simulink library "Renault_Library"  (Simulink 9.2)
settings: Solver=ode3  StartTime=0.0  StopTime=10.0  FixedStep=0.020  RelTol=1e-3  EnableMultiTasking=on
undefined symbols (not defined in the file or in its folder): ABC, AssystemProposal, b, chkval, CoeffA_C, CoeffB_C, CoeffMean_C, ConfTime_C, DiagDelay_C, DividePercent, FailureConfActive_C, FallingEdgeDuration_C, HighLevelThresholdVoltage_C, HighLevelVoltage_C, HighLevelVoltageTunable_C, InitValue_C, InputVoltRangeMax_C, InputVoltRangeMin_C, LowActiveContact_C, LowLevelThresholdVoltage_C, LowLevelVoltage_C, MaxOperatingVoltage_C, MinOperatingVoltage_C, mV2V_conversion, OUT1, OutOfRangeHigh_C, OutOfRangeLow_C, PinSupplySaturationMax, PinSupplySaturationMin, Res1Value_C, Res2Value_C, Res3Value_C, RisingEdgeDuration_C, RSA_CANSignalFailMonitoring, SampleTime_C, service14Table, service19Table, service22Table, service24Table, service28Table, service2ETable, service2FTable, service31Table, service87Table, SizeXStateDID2FTable, SizeXTable14, SizeXTable19, SizeXTable22, SizeXTable24, SizeXTable28, SizeXTable2E, SizeXTable2F, SizeXTable31, SizeXTable87, SizeYStateDID2FTable, SizeYTable14, SizeYTable19, SizeYTable22, SizeYTable24, SizeYTable28 ... (+22)
undefined symbols (not defined in the file): BatteryVoltage_ActivationThreshold_C, FrameStatus_AbsentValue_C, FrameStatus_ConfAbsentValue_C, MemThreshold_C, NAMEOFTHEMODEL_SAMPLE_TIME_CST, NbAbs1_C, NbConfAbs1_C, NbConfPres1_C, NbPres1_C, NOMDUSIGNAL_FaultConfTime_C, NOMDUSIGNAL_FaultRehabTime_C, NOMDUSIGNAL_switchvalue_C, NOMDUSIGNAL_unavailablevalue_C, PeriodFrame1_C, ResetTime_C, WakeUpTime_C
libraries: $bdroot x112, simulink x75
(part 7 of 32)

== system Renault_Library/Services ECU/HW Outputs ==
  note: DEFINED OUTPUTS
  note: FULLY TUNABLE OUTPUTS
  RSA_IHAB6Y : Subsystem  Tag=[LIB]  mask(RSA_IHAB6Y){LowLevelVoltage_C=500, InitValue_C=0, RisingEdgeDuration_C=0, FallingEdgeDuration_C=0, SampleTime_C=1, Tstab_C=30, Res1Value_C=100000, Res2Value_C=50000, Res3Value_C=50000}
      mask prompts: LowLevelVoltage_C "Low Level Voltage (mV)"; InitValue_C "Initial value of Output"; RisingEdgeDuration_C "Duration of the rising edge (ms)"; FallingEdgeDuration_C "Duration of the falling edge (ms)"; SampleTime_C "SampleTime : insert a sample time (ms)"; Tstab_C "Tstab : insert a stabilization waiting time (ms)"; Res1Value_C "Resistor 1 Value (Ohm)"; Res2Value_C "Resisitor 2 Value (Ohm)"; Res3Value_C "Resistor 3 Value (Ohm)"
      mask help:
      | IHAB.6.Y: High side Open collector (Y= 25 or 45mA)
      | Min Operating Voltage = 6 V
      | Max Operating Voltage = 16 V
      | High Level voltage = SupplyVoltage
      | Low Level Voltage = "to be parameterized"
      | Unit of ports for voltage are in millivolt (mV)
      |
      | Version : 01.01
      mask init:
      | MinOperatingVoltage_C=6000;
      | MaxOperatingVoltage_C= 16000; 
      | LowActiveContact_C=0; %1= LowActiveContact; 0= HighActiveContact
      | SampleTimeSec_C=SampleTime_C*.001; %unit conversion for sampletime
      mask Help:
      | IHAB.6.Y: High side Open collector (Y= 25 or 45mA)
      | Min Operating Voltage = 6 V
      | Max Operating Voltage = 16 V
      | High Level voltage = SupplyVoltage
      | Low Level Voltage = "to be parameterized"
      | Unit of ports for voltage are in millivolt (mV)
      | 
      | Version : 01.01
      mask Display: disp('IHAB_6_Y')
  RSA_IHABD6Y : Subsystem  Tag=[LIB]  mask(RSA_IHABD6Y){LowLevelVoltage_C=500, InitValue_C=0, RisingEdgeDuration_C=0, FallingEdgeDuration_C=0, SampleTime_C=1, Tstab_C=30, ConfTime_C=20, DiagDelay_C=30, VoffMaxUser_C=6000, VoffMinUser_C=1000, VonMaxUser_C=16000, VonMinUser_C=5000, Res1Value_C=100000, Res2Value_C=50000, Res3Value_C=50000}
      mask prompts: LowLevelVoltage_C "Low Level Voltage (mV)"; InitValue_C "Initial Value of Output"; RisingEdgeDuration_C "Duration of the rising edge (ms)"; FallingEdgeDuration_C "Duration of the falling edge (ms)"; SampleTime_C "SampleTime : insert a sample time (ms)"; Tstab_C "Tstab : insert a stabilization waiting time (ms)"; ConfTime_C "ConfTime: Fault Confirmation time (ms)"; DiagDelay_C "DiagDelay: Delay in (ms) to take into account input state"; VoffMaxUser_C "Threshold (mV) above which an SC+ failure is declared when T is OFF : Voff_max"; VoffMinUser_C "Threshold (mV) below which a RES SC- failure is declared when T is OFF: Voff_min"; VonMaxUser_C "Threshold (mV) above which an RES SC-/ SC+ failure is declared when T is ON : Von_max"; VonMinUser_C "Threshold (mV) below which an SC- failure is declared when T is ON :Von_min"; Res1Value_C "Resistor 1 Value (Ohm)"; Res2Value_C "Resisitor 2 Value (Ohm)"; Res3Value_C "Resistor 3 Value (Ohm)"
      mask help:
      | IHAB.D.6.Y: High side Open collector with OC diagnosis capability(Y=25 or 45mA)
      |
      | ECU output, active on high state. Link to one producer.
      | Min Operating Voltage = 6 V
      | Max Operating Voltage = 16 V
      | (Supply Voltage - coeff) < High Level voltage < SupplyVoltage
      | Low Level Voltage = "to be parametered"
      | Unit of ports for voltage are in millivolt (mV)
      |
      | 0.8V < Coeff < 2V following the value of SupplyVoltage
      |
      | Diagnosis:
      | Three failure states (SC+, SC-, OC), and two normal states (OKON and OKOFF) are possible.
      | The analogue thresholds to output these different states are tunable in diagnostic Parameters tab.
      |
      |    State Value      Diag voltage         Diagnostic States
      |      5              V > Voffmax                 SC+
      |      4         Voffmax > V > Voffmin            OKOFF
      |      3         Voffmin > V > Vonmax         Resistive SC-
      |      2          Vonmax > V > Vonmin             OKON
      |      1              V < Vonmin                  SC-
      |
      | Version : 01.01
      mask init:
      | MinOperatingVoltage_C= 6000;
      | MaxOperatingVoltage_C= 16000; 
      | LowActiveContact_C=0; %1= LowActiveContact; 0= HighActiveContact
      | SampleTimeSec_C=SampleTime_C*.001; %unit conversion for sampletime
      | FailureConfActive_C=1; %to enable the confirmation bloc (otherwise output remains to 0)
      | State_InfVonMin_C=1;
      | State_Von_C=2;
      | State_SupVonMax_C=3;
      | State_InfVoffMin_C=3;
      | State_Voff_C=4;
      | State_SupVoffMax_C=5;
      | CoeffCorr = Res3Value_C/(Res2Value_C+Res3Value_C);
      | VoffMax_C = VoffMaxUser_C*CoeffCorr;
      | VoffMin_C = VoffMinUser_C*CoeffCorr;
      | VonMax_C = VonMaxUser_C*CoeffCorr;
      | VonMin_C = VonMinUser_C*CoeffCorr;
      mask Help:
      | IHAB.D.6.Y: High side Open collector with OC diagnosis capability(Y=25 or 45mA)
      | 
      | ECU output, active on high state. Link to one producer.
      | Min Operating Voltage = 6 V
      | Max Operating Voltage = 16 V
      | (Supply Voltage - coeff) < High Level voltage < SupplyVoltage
      | Low Level Voltage = "to be parametered"
      | Unit of ports for voltage are in millivolt (mV)
      | 
      | 0.8V < Coeff < 2V following the value of SupplyVoltage
      | 
      | Diagnosis:
      | Three failure states (SC+, SC-, OC), and two normal states (OKON and OKOFF) are possible.
      | The analogue thresholds to output these different states are tunable in diagnostic Parameters tab.
      | 
      |    State Value      Diag voltage         Diagnostic States
      |      5              V > Voffmax                 SC+
      |      4         Voffmax > V > Voffmin            OKOFF
      |      3         Voffmin > V > Vonmax         Resistive SC-
      |      2          Vonmax > V > Vonmin             OKON
      |      1              V < Vonmin                  SC-
      | 
      | Version : 01.01
      mask Display: disp('IHAB_D_6_Y')
  RSA_IHABDXY : Subsystem  Tag=[LIB]  mask(RSA_IHABDXY){MinOperatingVoltage_C=0, MaxOperatingVoltage_C=0, LowLevelVoltage_C=500, InitValue_C=0, RisingEdgeDuration_C=0, FallingEdgeDuration_C=0, SampleTime_C=1, Tstab_C=30, ConfTime_C=20, DiagDelay_C=30, VoffMaxUser_C=6000, VoffMinUser_C=1000, VonMaxUser_C=16000, VonMinUser_C=5000, Res1Value_C=100000, Res2Value_C=50000, Res3Value_C=50000}
      mask prompts: MinOperatingVoltage_C "Min Operating Voltage (mV)"; MaxOperatingVoltage_C "Max Operating Voltage (mV)"; LowLevelVoltage_C "Low Level Voltage (mV)"; InitValue_C "Initial Value of Output"; RisingEdgeDuration_C "Duration of the rising edge (ms)"; FallingEdgeDuration_C "Duration of the falling edge (ms)"; SampleTime_C "SampleTime : insert a sample time (ms)"; Tstab_C "Tstab : insert a stabilization waiting time (ms)"; ConfTime_C "ConfTime: Fault Confirmation time (ms)"; DiagDelay_C "DiagDelay: Delay in (ms) to take into account input state"; VoffMaxUser_C "Threshold (mV) above which an SC+ failure is declared when T is OFF : Voff_max"; VoffMinUser_C "Threshold (mV) below which a RES SC- failure is declared when T is OFF: Voff_min"; VonMaxUser_C "Threshold (mV) above which an RES SC-/ SC+ failure is declared when T is ON : Von_max"; VonMinUser_C "Threshold (mV) below which an SC- failure is declared when T is ON :Von_min"; Res1Value_C "Resistor 1 Value (Ohm)"; Res2Value_C "Resisitor 2 Value (Ohm)"; Res3Value_C "Resistor 3 Value (Ohm)"
      mask help:
      | IHAB.D.X.Y: High side Open collector with OC diagnosis capability(Y=25 or 45mA)
      |
      | ECU output, active on high state. Link to one producer.
      | Working with a minimum supply voltage of X V.
      | (Supply Voltage - coeff) < High Level voltage < SupplyVoltage
      | Low Level Voltage = "to be parametered"
      | Unit of ports for voltage are in millivolt (mV)
      |
      | 0.8V < Coeff < 2V following the value of SupplyVoltage
      |
      | Diagnosis:
      | Three failure states (SC+, SC-, OC), and two normal states (OKON and OKOFF) are possible.
      | The analogue thresholds to output these different states are tunable in diagnostic Parameters tab.
      |
      |    State Value      Diag voltage         Diagnostic States
      |      5              V > Voffmax                 SC+
      |      4         Voffmax > V > Voffmin            OKOFF
      |      3         Voffmin > V > Vonmax         Resistive SC-
      |      2          Vonmax > V > Vonmin             OKON
      |      1              V < Vonmin                  SC-
      |
      | Version : 01.01
      mask init:
      | LowActiveContact_C=0; %1= LowActiveContact; 0= HighActiveContact
      | SampleTimeSec_C=SampleTime_C*.001; %unit conversion for sampletime
      | FailureConfActive_C=1; %to enable the confirmation bloc (otherwise output remains to 0)
      | State_InfVonMin_C=1;
      | State_Von_C=2;
      | State_SupVonMax_C=3;
      | State_InfVoffMin_C=3;
      | State_Voff_C=4;
      | State_SupVoffMax_C=5;
      | CoeffCorr = Res3Value_C/(Res2Value_C+Res3Value_C);
      | VoffMax_C = VoffMaxUser_C*CoeffCorr;
      | VoffMin_C = VoffMinUser_C*CoeffCorr;
      | VonMax_C = VonMaxUser_C*CoeffCorr;
      | VonMin_C = VonMinUser_C*CoeffCorr;
      mask Help:
      | IHAB.D.X.Y: High side Open collector with OC diagnosis capability(Y=25 or 45mA)
      | 
      | ECU output, active on high state. Link to one producer.
      | Working with a minimum supply voltage of X V.
      | (Supply Voltage - coeff) < High Level voltage < SupplyVoltage
      | Low Level Voltage = "to be parametered"
      | Unit of ports for voltage are in millivolt (mV)
      | 
      | 0.8V < Coeff < 2V following the value of SupplyVoltage
      | 
      | Diagnosis:
      | Three failure states (SC+, SC-, OC), and two normal states (OKON and OKOFF) are possible.
      | The analogue thresholds to output these different states are tunable in diagnostic Parameters tab.
      | 
      |    State Value      Diag voltage         Diagnostic States
      |      5              V > Voffmax                 SC+
      |      4         Voffmax > V > Voffmin            OKOFF
      |      3         Voffmin > V > Vonmax         Resistive SC-
      |      2          Vonmax > V > Vonmin             OKON
      |      1              V < Vonmin                  SC-
      | 
      | Version : 01.01
      mask Display: disp('IHAB_D_X_Y')
  RSA_ILAB6Y : Subsystem  Tag=[LIB]  mask(RSA_ILAB6Y){LowLevelVoltage_C=500, InitValue_C=0, RisingEdgeDuration_C=0, FallingEdgeDuration_C=0, SampleTime_C=1, Tstab_C=30}
      mask prompts: LowLevelVoltage_C "Low Level Voltage (mV)"; InitValue_C "Initial Value of Output"; RisingEdgeDuration_C "Duration of the rising edge (ms)"; FallingEdgeDuration_C "Duration of the falling edge (ms)"; SampleTime_C "SampleTime : insert a sample time (ms)"; Tstab_C "Tstab : insert a stabilization waiting time (ms)"
      mask help:
      | ILAB.6.Y: Low side Open collector(Y=25 or 45mA)
      | Min Operating Voltage = 6 V
      | Max Operating Voltage = 16 V
      | High Level voltage = SupplyVoltage
      | Low Level Voltage = "to be parameterized"
      | Unit of ports for voltage are in millivolt (mV)
      |
      | Version : 01.01
      mask init:
      | MinOperatingVoltage_C=6000;
      | MaxOperatingVoltage_C= 16000;
      | HighLevelVoltage_C=0;
      | HighLevelVoltageTunable_C=0;
      | LowActiveContact_C=1; %1= LowActiveContact; 0= HighActiveContact
      | SampleTimeSec_C=SampleTime_C*.001; %unit conversion for sampletime
      mask Help:
      | ILAB.6.Y: Low side Open collector(Y=25 or 45mA)
      | Min Operating Voltage = 6 V
      | Max Operating Voltage = 16 V
      | High Level voltage = SupplyVoltage
      | Low Level Voltage = "to be parameterized"
      | 
      | Version : 01.01
      mask Display: disp('ILAB_6_Y')
  RSA_ILAB8250REL : Subsystem  Tag=[LIB]  mask(RSA_ILAB8250REL){LowLevelVoltage_C=500, InitValue_C=0, RisingEdgeDuration_C=0, FallingEdgeDuration_C=0, SampleTime_C=1, Tstab_C=30, Res2Value_C=50000, Res3Value_C=50000}
      mask prompts: LowLevelVoltage_C "Low Level Voltage (mV)"; InitValue_C "Initial Value of Output"; RisingEdgeDuration_C "Duration of the rising edge (ms)"; FallingEdgeDuration_C "Duration of the falling edge (ms)"; SampleTime_C "SampleTime : insert a sample time (ms)"; Tstab_C "Tstab : insert a stabilization waiting time (ms)"; Res2Value_C "Resistor 2 Value (Ohm)"; Res3Value_C "Resistor 3 Value (Ohm)"
      mask help:
      | ILAB.8.250.REL: Low side Open collector for Relay control.
      |
      | This output will drive a relay with resistor OR a relay with a diode but NOT BOTH at the same time.
      | ECU output, active on low state. Link to one producer.
      | Min Operating Voltage = 8 V
      | Max Operating Voltage = 16 V
      | High Level voltage = SupplyVoltage
      | Low Level Voltage = "to be parameterized"
      | Unit of ports for voltage are in millivolt (mV)
      |
      | Version : 01.01
      mask init:
      | MinOperatingVoltage_C=8000;
      | MaxOperatingVoltage_C=16000;
      | HighLevelVoltage_C=0;
      | HighLevelVoltageTunable_C=0;
      | LowActiveContact_C=1; %1= LowActiveContact; 0= HighActiveContact
      | SampleTimeSec_C=SampleTime_C*.001; %unit conversion for sampletime
      mask Help:
      | ILAB.8.250.REL: Low side Open collector for Relay control.
      | 
      | This output will drive a relay with resistor OR a relay with a diode but NOT BOTH at the same time.
      | ECU output, active on low state. Link to one producer.
      | Min Operating Voltage = 8 V
      | Max Operating Voltage = 16 V
      | High Level voltage = SupplyVoltage
      | Low Level Voltage = "to be parameterized"
      | Unit of ports for voltage are in millivolt (mV)
      | 
      | Version : 01.01
      mask Display: disp('ILAB_8_250_REL')
  RSA_ILABD6Y : Subsystem  Tag=[LIB]  mask(RSA_ILABD6Y){LowLevelVoltage_C=500, InitValue_C=0, RisingEdgeDuration_C=0, FallingEdgeDuration_C=0, SampleTime_C=1, Tstab_C=30, ConfTime_C=20, DiagDelay_C=30, VoffMaxUser_C=6000, VoffMinUser_C=1000, VonMaxUser_C=16000, VonMinUser_C=5000, Res1Value_C=100000, Res2Value_C=50000, Res3Value_C=50000}
      mask prompts: LowLevelVoltage_C "Low Level Voltage (mV)"; InitValue_C "Initial Value of Output"; RisingEdgeDuration_C "Duration of the rising edge (ms)"; FallingEdgeDuration_C "Duration of the falling edge (ms)"; SampleTime_C "SampleTime : insert a sample time (ms)"; Tstab_C "Tstab : insert a stabilization waiting time (ms)"; ConfTime_C "ConfTime: Fault Confirmation time (ms)"; DiagDelay_C "DiagDelay: Delay in (ms) to take into account input state"; VoffMaxUser_C "Threshold (mV) above which an SC+ failure is declared when T is OFF : Voff_max"; VoffMinUser_C "Threshold (mV) below which a RES SC- failure is declared when T is OFF: Voff_min"; VonMaxUser_C "Threshold (mV) above which an RES SC-/ SC+ failure is declared when T is ON : Von_max"; VonMinUser_C "Threshold (mV) below which an SC- failure is declared when T is ON :Von_min"; Res1Value_C "Resistor 1 Value (Ohm)"; Res2Value_C "Resisitor 2 Value (Ohm)"; Res3Value_C "Resistor 3 Value (Ohm)"
      mask help:
      | ILAB.D.6.Y: Low side Open collector with OC diagnosis capability(Y=25 or 45mA)
      |
      | ECU output, active on low state. Link to one producer.
      | Min Operating Voltage = 6 V
      | Max Operating Voltage = 16 V
      | High Level voltage = SupplyVoltage
      | Low Level Voltage = "to be parameterized"
      | Unit of ports for voltage are in millivolt (mV)
      |
      | Diagnosis:
      | Three failure states (SC+, SC-, OC), and two normal states (OKON and OKOFF) are possible.
      | The analogue thresholds to output these different states are tunable in diagnostic Parameters tab.
      |
      |    State Value      Diag voltage         Diagnostic States
      |      5              V > Voffmax                 SC+
      |      4         Voffmax > V > Voffmin            OKOFF
      |      3         Voffmin > V > Vonmax         Resistive SC-
      |      2          Vonmax > V > Vonmin             OKON
      |      1              V < Vonmin                  SC-
      |
      | Version : 01.01
      mask init:
      | MinOperatingVoltage_C=6000;
      | MaxOperatingVoltage_C=16000; 
      | HighLevelVoltage_C=0;
      | HighLevelVoltageTunable_C=0;
      | LowActiveContact_C=1; %1= LowActiveContact; 0= HighActiveContact
      | SampleTimeSec_C=SampleTime_C*.001; %unit conversion for sampletime
      | FailureConfActive_C=1; %to enable the confirmation bloc (otherwise output remains to 0)
      | State_InfVonMin_C=1;
      | State_Von_C=2;
      | State_SupVonMax_C=3;
      | State_InfVoffMin_C=3;
      | State_Voff_C=4;
      | State_SupVoffMax_C=5;
      | CoeffCorr = Res3Value_C/(Res2Value_C+Res3Value_C);
      | VoffMax_C = VoffMaxUser_C*CoeffCorr;
      | VoffMin_C = VoffMinUser_C*CoeffCorr;
      | VonMax_C = VonMaxUser_C*CoeffCorr;
      | VonMin_C = VonMinUser_C*CoeffCorr;
      mask Help:
      | ILAB.D.6.Y: Low side Open collector with OC diagnosis capability(Y=25 or 45mA)
      | 
      | ECU output, active on low state. Link to one producer.
      | Min Operating Voltage = 6 V
      | Max Operating Voltage = 16 V
      | High Level voltage = SupplyVoltage
      | Low Level Voltage = "to be parameterized"
      | Unit of ports for voltage are in millivolt (mV)
      | 
      | Diagnosis:
      | Three failure states (SC+, SC-, OC), and two normal states (OKON and OKOFF) are possible.
      | The analogue thresholds to output these different states are tunable in diagnostic Parameters tab.
      | 
      |    State Value      Diag voltage         Diagnostic States
      |      5              V > Voffmax                 SC+
      |      4         Voffmax > V > Voffmin            OKOFF
      |      3         Voffmin > V > Vonmax         Resistive SC-
      |      2          Vonmax > V > Vonmin             OKON
      |      1              V < Vonmin                  SC-
      | 
      | Version : 01.01
      mask Display: disp('ILAB_D_6_Y')
  RSA_ILABD8250REL : Subsystem  Tag=[LIB]  mask(RSA_ILABD8250REL){LowLevelVoltage_C=500, InitValue_C=0, RisingEdgeDuration_C=0, FallingEdgeDuration_C=0, SampleTime_C=1, Tstab_C=30, ConfTime_C=20, DiagDelay_C=30, VoffMaxUser_C=16000, VoffMinUser_C=500, VonMaxUser_C=1000, VonMinUser_C=10, Res2Value_C=50000, Res3Value_C=50000}
      mask prompts: LowLevelVoltage_C "Low Level Voltage (mV)"; InitValue_C "Initial Value of Output"; RisingEdgeDuration_C "Duration of the rising edge (ms)"; FallingEdgeDuration_C "Duration of the falling edge (ms)"; SampleTime_C "SampleTime : insert a sample time (ms)"; Tstab_C "Tstab : insert a stabilization waiting time (ms)"; ConfTime_C "ConfTime: Fault Confirmation time (ms)"; DiagDelay_C "DiagDelay: Delay in (ms) to take into account input state"; VoffMaxUser_C "Threshold (mV) above which an SC+ failure is declared when T is OFF : Voff_max"; VoffMinUser_C "Threshold (mV) below which a RES SC- failure is declared when T is OFF: Voff_min"; VonMaxUser_C "Threshold (mV) above which an RES SC-/ SC+ failure is declared when T is ON : Von_max"; VonMinUser_C "Threshold (mV) below which an SC- failure is declared when T is ON :Von_min"; Res2Value_C "Resistor 2 Value (Ohm)"; Res3Value_C "Resistor 3 Value (Ohm)"
      mask help:
      | ILAB.8.250.REL: Low side Open collector for Relay control.
      |
      | This output will drive a relay with resistor OR a relay with a diode but NOT BOTH at the same time.
      | ECU output, active on low state. Link to one producer.
      | Min Operating Voltage = 8 V
      | Max Operating Voltage = 16 V
      | High Level voltage = SupplyVoltage
      | Low Level Voltage = "to be parameterized"
      | Unit of ports for voltage are in millivolt (mV)
      |
      | Diagnosis:
      | Three failure states (SC+, SC-, OC), and two normal states (OKON and OKOFF) are possible.
      | The analogue thresholds to output these different states are tunable in diagnostic Parameters tab.
      |
      |    State             T=OFF               T=ON               Diagnostic States
      |      5              V > Voffmax                                    SC+
      |      4         Voffmin < V < Voffmax                              OKOFF
      |      3         Vonmax < V < Voffmin                               SC-
      |      2                              Vonmin < V < Vonmax           OKON
      |      1                                  V < Vonmin                 SC-
      |
      | Version : 01.01
      mask init:
      | MinOperatingVoltage_C=8000;
      | MaxOperatingVoltage_C=16000;
      | HighLevelVoltage_C=0;
      | HighLevelVoltageTunable_C=0;
      | LowActiveContact_C=1; %1= LowActiveContact; 0= HighActiveContact
      | SampleTimeSec_C=SampleTime_C*.001; %unit conversion for sampletime
      | FailureConfActive_C=1; %to enable the confirmation bloc (otherwise output remains to 0)
      | State_InfVonMin_C=1;
      | State_Von_C=2;
      | State_SupVonMax_C=3;
      | State_InfVoffMin_C=3;
      | State_Voff_C=4;
      | State_SupVoffMax_C=5;
      | CoeffCorr = Res3Value_C/(Res2Value_C+Res3Value_C);
      | VoffMax_C = VoffMaxUser_C*CoeffCorr;
      | VoffMin_C = VoffMinUser_C*CoeffCorr;
      | VonMax_C = VonMaxUser_C*CoeffCorr;
      | VonMin_C = VonMinUser_C*CoeffCorr;
      mask Help:
      | ILAB.8.250.REL: Low side Open collector for Relay control.
      | 
      | This output will drive a relay with resistor OR a relay with a diode but NOT BOTH at the same time.
      | ECU output, active on low state. Link to one producer.
      | Min Operating Voltage = 8 V
      | Max Operating Voltage = 16 V
      | High Level voltage = SupplyVoltage
      | Low Level Voltage = "to be parameterized"
      | Unit of ports for voltage are in millivolt (mV)
      | 
      | Diagnosis:
      | Three failure states (SC+, SC-, OC), and two normal states (OKON and OKOFF) are possible.
      | The analogue thresholds to output these different states are tunable in diagnostic Parameters tab.
      | 
      |    State             T=OFF               T=ON               Diagnostic States
      |      5              V > Voffmax                                    SC+
      |      4         Voffmin < V < Voffmax                              OKOFF
      |      3         Vonmax < V < Voffmin                               SC-
      |      2                              Vonmin < V < Vonmax           OKON
      |      1                                  V < Vonmin                 SC-
      | 
      | Version : 01.01
      mask Display: disp('ILAB_D_8_250_REL')
  RSA_ILABDXY : Subsystem  Tag=[LIB]  mask(RSA_ILABDXY){MinOperatingVoltage_C=0, MaxOperatingVoltage_C=0, LowLevelVoltage_C=500, HighLevelVoltage_C=0, HighLevelVoltageTunable_C=0, InitValue_C=0, RisingEdgeDuration_C=0, FallingEdgeDuration_C=0, SampleTime_C=1, Tstab_C=30, ConfTime_C=20, DiagDelay_C=30, VoffMaxUser_C=6000, VoffMinUser_C=1000, VonMaxUser_C=16000, VonMinUser_C=5000, Res1Value_C=100000, Res2Value_C=50000, Res3Value_C=50000}
      mask prompts: MinOperatingVoltage_C "Min Operating Voltage (mV)"; MaxOperatingVoltage_C "Max Operating Voltage (mV)"; LowLevelVoltage_C "Low Level Voltage (mV)"; HighLevelVoltage_C "High Level Voltage (mV)"; HighLevelVoltageTunable_C "High Level Voltage Tunable (=0 to have HighLevelVoltage=SupplyVoltage; =1 to use HighLevelVoltage parameter above)"; InitValue_C "Initial Value of Output"; RisingEdgeDuration_C "Duration of the rising edge (ms)"; FallingEdgeDuration_C "Duration of the falling edge (ms)"; SampleTime_C "SampleTime : insert a sample time (ms)"; Tstab_C "Tstab : insert a stabilization waiting time (ms)"; ConfTime_C "ConfTime: Fault Confirmation time (ms)"; DiagDelay_C "DiagDelay: Delay in (ms) to take into account input state"; VoffMaxUser_C "Threshold (mV) above which an SC+ failure is declared when T is OFF : Voff_max"; VoffMinUser_C "Threshold (mV) below which a RES SC- failure is declared when T is OFF: Voff_min"; VonMaxUser_C "Threshold (mV) above which an RES SC-/ SC+ failure is declared when T is ON : Von_max"; VonMinUser_C "Threshold (mV) below which an SC- failure is declared when T is ON :Von_min"; Res1Value_C "Resistor 1 Value (Ohm)"; Res2Value_C "Resisitor 2 Value (Ohm)"; Res3Value_C "Resistor 3 Value (Ohm)"
      mask help:
      | ILAB.D.X.Y: Low side Open collector with OC diagnosis capability(Y=25 or 45mA)
      |
      | ECU output, active on low state. Link to one producer.
      | Working with a minimum supply voltage of X V.
      | High Level voltage = "to be parameterized" if tunable=1, =SupplyVoltage if tunable=0
      | Low Level Voltage = "to be parameterized"
      | Unit of ports for voltage are in millivolt (mV)
      |
      | Diagnosis:
      | Three failure states (SC+, SC-, OC), and two normal states (OKON and OKOFF) are possible.
      | The analogue thresholds to output these different states are tunable in diagnostic Parameters tab.
      |
      |    State Value      Diag voltage         Diagnostic States
      |      5              V > Voffmax                 SC+
      |      4         Voffmax > V > Voffmin            OKOFF
      |      3         Voffmin > V > Vonmax         Resistive SC-
      |      2          Vonmax > V > Vonmin             OKON
      |      1              V < Vonmin                  SC-
      |
      | Version : 01.01
      mask init:
      | HighLevelVoltageTunable_C=0;
      | LowActiveContact_C=1; %1= LowActiveContact; 0= HighActiveContact
      | SampleTimeSec_C=SampleTime_C*.001; %unit conversion for sampletime
      | FailureConfActive_C=1; %to enable the confirmation bloc (otherwise output remains to 0)
      | State_InfVonMin_C=1;
      | State_Von_C=2;
      | State_SupVonMax_C=3;
      | State_InfVoffMin_C=3;
      | State_Voff_C=4;
      | State_SupVoffMax_C=5;
      | CoeffCorr = Res3Value_C/(Res2Value_C+Res3Value_C);
      | VoffMax_C = VoffMaxUser_C*CoeffCorr;
      | VoffMin_C = VoffMinUser_C*CoeffCorr;
      | VonMax_C = VonMaxUser_C*CoeffCorr;
      | VonMin_C = VonMinUser_C*CoeffCorr;
      mask Help:
      | ILAB.D.X.Y: Low side Open collector with OC diagnosis capability(Y=25 or 45mA)
      | 
      | ECU output, active on low state. Link to one producer.
      | Working with a minimum supply voltage of X V.
      | High Level voltage = "to be parameterized" if tunable=1, =SupplyVoltage if tunable=0
      | Low Level Voltage = "to be parameterized"
      | Unit of ports for voltage are in millivolt (mV)
      | 
      | Diagnosis:
      | Three failure states (SC+, SC-, OC), and two normal states (OKON and OKOFF) are possible.
      | The analogue thresholds to output these different states are tunable in diagnostic Parameters tab.
      | 
      |    State Value      Diag voltage         Diagnostic States
      |      5              V > Voffmax                 SC+
      |      4         Voffmax > V > Voffmin            OKOFF
      |      3         Voffmin > V > Vonmax         Resistive SC-
      |      2          Vonmax > V > Vonmin             OKON
      |      1              V < Vonmin                  SC-
      | 
      | Version : 01.01
      mask Display: disp('ILAB_D_X_Y')
  RSA_ILCD6Y : Subsystem  Tag=[LIB]  mask(RSA_ILCD6Y){LowLevelVoltage_C=500, InitValue_C=0, RisingEdgeDuration_C=0, FallingEdgeDuration_C=0, SampleTime_C=1, Tstab_C=30, Res1Value_C=100000, Res2Value_C=50000, Res3Value_C=50000}
      mask prompts: LowLevelVoltage_C "Low Level Voltage (mV)"; InitValue_C "Initial Value of Output"; RisingEdgeDuration_C "Duration of the rising edge (ms)"; FallingEdgeDuration_C "Duration of the falling edge (ms)"; SampleTime_C "SampleTime : insert a sample time (ms)"; Tstab_C "Tstab : insert a stabilization waiting time (ms)"; Res1Value_C "Resistor 1 Value (Ohm)"; Res2Value_C "Resisitor 2 Value (Ohm)"; Res3Value_C "Resistor 3 Value (Ohm)"
      mask help:
      | ILCD.6.Y: Low side Open collector, with pull-up for bidirectional
      | communication.(Y=25 or 45mA).
      |
      | ECU output, active on low state. Link to one producer.
      | Min Operating Voltage = 6 V
      | Max Operating Voltage = 16 V
      | High Level voltage = SupplyVoltage
      | Low Level Voltage = "to be parameterized"
      | Unit of ports for voltage are in millivolt (mV)
      |
      | should be used for bidirectional data exchange.
      | Internal Feedback to connect to ILB Input.
      |
      | Version : 01.01
      mask init:
      | MinOperatingVoltage_C=6000;
      | MaxOperatingVoltage_C=16000; 
      | HighLevelVoltage_C=0;
      | HighLevelVoltageTunable_C=0;
      | LowActiveContact_C=1; %1= LowActiveContact; 0= HighActiveContact
      | SampleTimeSec_C=SampleTime_C*.001; %unit conversion for sampletime
      | FailureConfActive_C=1; %to enable the confirmation bloc (otherwise output remains to 0)
      | State_InfVonMin_C=1;
      | State_Von_C=2;
      | State_SupVonMax_C=3;
      | State_InfVoffMin_C=3;
      | State_Voff_C=4;
      | State_SupVoffMax_C=5;
      | VoffMin_C=1000;
      | VoffMax_C=6000;
      | VonMin_C=5000;
      | VonMax_C=16000;
      | DiagDelay_C = 0;
      | ConfTime_C = 20;
      mask Help:
      | ILCD.6.Y: Low side Open collector, with pull-up for bidirectional
      | communication.(Y=25 or 45mA).
      | 
      | ECU output, active on low state. Link to one producer.
      | Min Operating Voltage = 6 V
      | Max Operating Voltage = 16 V
      | High Level voltage = SupplyVoltage
      | Low Level Voltage = "to be parameterized"
      | Unit of ports for voltage are in millivolt (mV)
      | 
      | should be used for bidirectional data exchange.
      | Internal Feedback to connect to ILB Input.
      | 
      | Version : 01.01
      mask Display: disp('ILCD_6_Y')
  RSA_IZCD545TTL : Subsystem  Tag=[LIB]  mask(RSA_IZCD545TTL){InitValue_C=0, SampleTime_C=1, Tstab_C=30}
      mask prompts: InitValue_C "Initial Value of Output"; SampleTime_C "SampleTime : insert a sample time (ms)"; Tstab_C "Tstab : insert a stabilization waiting time (ms)"
      mask help:
      | IZCD.5.45.TTL:
      | Push-Pull Output
      | Min Operating Voltage = 4.5 V
      | Max Operating Voltage = 5.5 V
      | High Level voltage = 2.4 V
      | Low Level Voltage = 0.4 V
      | Unit of ports for voltage are in millivolt (mV)
      |
      | Version : 01.01
      mask init:
      | MinOperatingVoltage_C=4500;
      | MaxOperatingVoltage_C=5500;
      | LowLevelVoltage_C=400;
      | HighLevelVoltage_C=2400;
      | HighLevelVoltageTunable_C=1;
      | LowActiveContact_C=0; %1= LowActiveContact; 0= HighActiveContact
      | SampleTimeSec_C=SampleTime_C*.001; %unit conversion for sampletime
      | FallingEdgeDuration_C = 0;
      | RisingEdgeDuration_C = 0;
      mask Help:
      | IZCD.5.45.TTL:
      | Push-Pull Output
      | Min Operating Voltage = 4.5 V
      | Max Operating Voltage = 5.5 V
      | High Level voltage = 2.4 V
      | Low Level Voltage = 0.4 V
      | Unit of ports for voltage are in millivolt (mV)
      | 
      | Version : 01.01
      mask Display: disp('IZCD_5_45_TTL')
  RSA_SmartFET : Subsystem  Tag=[LIB]  mask(RSA_SmartFET){MinOperatingVoltage_C=6000, MaxOperatingVoltage_C=16000}
      mask prompts: MinOperatingVoltage_C "Min Operating Voltage (mV)"; MaxOperatingVoltage_C "Max Operating Voltage (mV)"
      mask help:
      | RSA_SmartFET
      |
      | Operating Voltage Min and Max can be configured on mask
      | Voltages are in mV
      |
      | if NoSmartProtection=0 then PhysicalOutput=0
      | OutputCmd=0;
      | else PhysicalOutput=(PwmRatio/100)*PinSupply
      | OutputCmd=PwmRatio
      |
      | Version : 01.00
      mask init:
      | DividePercent= 100;
      | SmartProtectionOn_Zero= 0;
      | mV2V_conversion = .001;
      | PinSupplySaturationMax = 20;
      | PinSupplySaturationMin = .1;
      mask Help:
      | RSA_SmartFET
      | 
      | Operating Voltage Min and Max can be configured on mask
      | Voltages are in mV
      | 
      | if NoSmartProtection=0 then PhysicalOutput=0
      | OutputCmd=0;
      | else PhysicalOutput=(PwmRatio/100)*PinSupply
      | OutputCmd=PwmRatio
      | 
      | Version : 01.00
      mask Display: disp('SmartFET')

== system Renault_Library/Services ECU/Mux ==
  note: Mux
  RSA_CANFaultManagement : Subsystem  Tag=[LIB]  mask(RSA_CANFaultManagement){CANSignal_SwitchValue=NOMDUSIGNAL_switchvalue_C, CANSignal_UnavailableValue=NOMDUSIGNAL_unavailablevalue_C, Frame_AbsentValue=FrameStatus_AbsentValue_C, Frame_ConfirmedAbsentValue=FrameStatus_ConfAbsentValue_C, CANSignal_FaultConfTime=NOMDUSIGNAL_FaultConfTime_C, CANSignal_FaultRehabTime=NOMDUSIGNAL_FaultRehabTime_C}
      mask prompts: CANSignal_SwitchValue "Value of the switch for using Invalid (0) or Last Value (1)"; CANSignal_UnavailableValue "Value of unavailable signal"; Frame_AbsentValue "Value of FrameStatus when absent"; Frame_ConfirmedAbsentValue "Value of FrameStatus when ConfAbsent"; CANSignal_FaultConfTime "FaultConfirmation_Time (s)"; CANSignal_FaultRehabTime "FaultRehab_Time (s)"
      mask help:
      | Subsystem which output failure value when the frame is confirmed absent.
      | It can output either an invalid value or held the last value.
      | Signal_Unknown and Signal_Unknown_Confirmed are boolean flags to re-use in model, equals to "1" when the corresponding state is right.
      |
      | Inputs overview:
      | Fault_Management_Allowed can be linked to ECU_Activation or GADE_Activation following the maturity of the model
      | Failure shall be a boolean equals to '1' when a failure occures.
      | Signal is the current value of the signal.
      | Frame_Status corresponds to output of RSA_FrameMonitoring
      | ClearDiagnosticInformation is to reset the current states.
      |
      | Version : 01.00
      mask Help:
      | Subsystem which output failure value when the frame is confirmed absent.
      | It can output either an invalid value or held the last value.
      | Signal_Unknown and Signal_Unknown_Confirmed are boolean flags to re-use in model, equals to "1" when the corresponding state is right.
      | 
      | Inputs overview:
      | Fault_Management_Allowed can be linked to ECU_Activation or GADE_Activation following the maturity of the model
      | Failure shall be a boolean equals to '1' when a failure occures.
      | Signal is the current value of the signal.
      | Frame_Status corresponds to output of RSA_FrameMonitoring
      | ClearDiagnosticInformation is to reset the current states.
      | 
      | Version : 01.00
      mask Display: disp('CAN Fault Management')
  RSA_CANSignalFailMonitoring : Subsystem  Tag=[LIB]  mask(RSA_CANSignalFailMonitoring){ResetValue=0, Ts=SampleTime_C}
      mask prompts: ResetValue "Reset Value"; Ts "SampleTime (s)"
      mask help:
      | Subsystem to confirm failure on signal
      |
      | Inputs:
      | Fault_Management_Allowed (boolean)
      | ClearDiagnosticInformation = reset diag
      | Failure= boolean true when a failure occures
      | Rehab= boolean true when the failure disappears
      | FaultConfirmation_Time = duration of failure to confirm the failure
      | FaultRehab_Time = duration of disappearance to confirm the rehabilitation
      |
      | Output:
      | Failure_flag (boolean) = true if failure confirmed/ false if rehab
      |
      | Version : 01.00
      mask Help:
      | Inputs:
      | Fault_Management_Allowed (boolean)
      | ClearDiagnosticInformation = reset diag
      | Failure= boolean true when a failure occures
      | Rehab= boolean true when the failure disappears
      | FaultConfirmation_Time = duration of failure to confirm the failure
      | FaultRehab_Time = duration of disappearance to confirm the rehabilitation
      | 
      | Output:
      | Failure_flag (boolean) = true if failure confirmed/ false if rehab
      | 
      | Version : 01.00
  RSA_ConvertFromMux : Subsystem  Tag=[LIB]  mask(RSA_ConvertFromMux){Factor=1, Offset=0, Divisor=1, Unavailable=0}
      mask prompts: Unavailable "Unavailable Value"
      mask help:
      | Convert a Mux Signal to a model data.
      |
      | Y: Model Data
      | X: Mux Signal
      |
      | Y = (Factor * X + Offset)/Divisor
      |
      | Version : 01.00
      mask Help:
      | Convert a Mux Signal to a model data.
      | 
      | Y: Model Data
      | X: Mux Signal
      | 
      | Y = (Factor * X + Offset)/Divisor
      | 
      | Version : 01.00
      mask Display:
      | disp('Convert From Mux')
      | port_label('Input',1,'SIGNAL')
      | port_label('Output',1,'DATA')
      | port_label('Output',2,'UNAVAILABLE_SIGNAL')
  RSA_ConvertToMux : Subsystem  Tag=[LIB]  mask(RSA_ConvertToMux){Factor=1, Offset=0, Divisor=1}
      mask help:
      | Convert a model data to a Mux Signal.
      |
      | Y: Mux Signal
      | X: Model Data
      |
      | Y = (Factor * X - Offset)/Divisor
      |
      | Version : 01.00
      mask Help:
      | Convert a model data to a Mux Signal.
      | 
      | Y: Mux Signal
      | X: Model Data
      | 
      | Y = (Factor * X - Offset)/Divisor
      | 
      | Version : 01.00
      mask Display:
      | disp('Convert To Mux')
      | port_label('Input',1,'DATA')
      | port_label('Output',1,'SIGNAL')
  RSA_FrameMonitoring : Subsystem  Tag=[LIB]  mask(RSA_FrameMonitoring){}
      mask help:
      | Use to monitoring a frame from CAN.
      | Outpout is a counter which is incremented each time a frame is received (i.e change of the input)
      | Output counter values are 0,1,2,3
      |
      | Version : 01.00
      mask init:
      | MAX_COUNTER = 4;
      | MIN_COUNTER = 0;
      | INCREMENT_COUNTER = 1;
      mask Help:
      | Use to monitoring a frame from CAN.
      | Outpout is a counter which is incremented each time a frame is received (i.e change of the input)
      | Output counter values are 0,1,2,3
      | 
      | Version : 01.00
      mask Display:
      | disp('Frame Monitoring')
      | port_label('Input',1,'FRAME_RECEIVED')
      | port_label('Output',1,'FRAME_SEND')
  RSA_FrameStatusMgt : Subsystem  Tag=[LIB]  mask(RSA_FrameStatusMgt){PeriodCalib=PeriodFrame1_C, SampleTimeCalib=SampleTime_C, NbAbsCalib=NbAbs1_C, NbConfCalib=NbConfAbs1_C, NbPresCalib=NbPres1_C, NbConfPresCalib=NbConfPres1_C, ToleranceCalib=10}
      mask prompts: PeriodCalib "Period of Frame (s)"; SampleTimeCalib "Sample Time (s)"; NbAbsCalib "Number of Period to declare an Absent Frame"; NbConfCalib "Number of Period to confirm an Absent Frame"; NbPresCalib "Number of Period to declare a Present Frame"; NbConfPresCalib "Number of Period to confirm a Present Frame"; ToleranceCalib "TimeOut to declare an absent frame (%)"
      mask help:
      | Input "Fault_Management_Allowed" to be linked to ECU Activation OR GADE Activation
      |
      | Frame_Update = 1 each time the Frame counter is incrementing
      | Frame_Status gives various states as following:
      | = 1 for normally absent (absent but not expected) and init
      | = 2 for present
      | = 3 for anormally absent (absent and expected)
      | = 4 for anormally absent confirmed (absent and expected confirmed)
      |
      | A delay of NbAbs*Period*TimeOut is waited to declare an absent frame
      | A delay of NbConfAbs*Period*TimeOut is waited to confirm an absent frame
      | If NbConfPres = 0, there is no delay waited to rehabilite the frame
      | If NbPres = 0, stateflow is continuously waiting (without going back to ConfirmedAbsente State)
      | a NbConfPres Time to rehabilite the frame
      | NbAbs, NbConf, Period and Tt are configurable parameters
      |
      | Version : 01.00
      mask init:
      | % Init value for Stateflow
      | Frame_Absent_Not_Expected=1;
      | Frame_Present=2;
      | Frame_Absent=3;
      | Frame_Confirmed_Absent=4;
      | 
      | ToleranceFactor=1+ToleranceCalib*.01;
      mask Help:
      | Input "Fault_Management_Allowed" to be linked to ECU Activation OR GADE Activation
      | 
      | Frame_Update = 1 each time the Frame counter is incrementing
      | Frame_Status gives various states as following:
      | = 1 for normally absent (absent but not expected) and init
      | = 2 for present
      | = 3 for anormally absent (absent and expected)
      | = 4 for anormally absent confirmed (absent and expected confirmed)
      | 
      | A delay of NbAbs*Period*TimeOut is waited to declare an absent frame
      | A delay of NbConfAbs*Period*TimeOut is waited to confirm an absent frame
      | If NbConfPres = 0, there is no delay waited to rehabilite the frame
      | If NbPres = 0, stateflow is continuously waiting (without going back to ConfirmedAbsente State)
      | a NbConfPres Time to rehabilite the frame
      | NbAbs, NbConf, Period and Tt are configurable parameters
      | 
      | Version : 01.00
      mask Display: disp('Frame status mgt')
  RSA_FrameUpdate : Subsystem  Tag=[LIB]  mask(RSA_FrameUpdate){FrameUpdateAlwaysRunning=on}
      mask prompts: FrameUpdateAlwaysRunning "Boolean for having FrameUpdate always running even if ECU or GADE off (checked =1 for always running)"
      mask help:
      | This block should be placed in the LLS/ D-EIPF interface.
      | Frame_Update = 1 each time the counter Frame_RX is incrementing.
      |
      | Version : 01.00
      mask Help:
      | This block should be placed in the LLS/ D-EIPF interface.
      | Frame_Update = 1 each time the counter Frame_RX is incrementing.
      | 
      | Version : 01.00
      mask Display: disp('Frame Update')
  RSA_MuxMonitoring : Subsystem  Tag=[LIB]  mask(RSA_MuxMonitoring){FRAME_PERIOD=100, CONFIRMED_ABSENT_PERIOD=2000}
      mask prompts: FRAME_PERIOD "Period (ms)"; CONFIRMED_ABSENT_PERIOD "Confirmed absent (ms)"
      mask help:
      | Use to monitoring CAN frame.
      | - Period: Frame period (in ms)
      | - Confirmed absent period: Time to confirmed the absence of frame (in ms)
      | Version : 01.00
      mask Help:
      | Use to monitoring CAN frame.
      | - Period: Frame period (in ms)
      | - Confirmed absent period: Time to confirmed the absence of frame (in ms)
      | Version : 01.00
      mask Display:
      | disp('Mux Monitoring')
      | port_label('Input',1,'FRAME_RECEIVED')
      | port_label('Output',1,'FRAME_STATUS')
      | port_label('Output',2,'FRAME_PRESENT')
  RSA_MuxSendFrame : Subsystem  Tag=[LIB]  mask(RSA_MuxSendFrame){FRAME_PERIOD=100, MAX_PARAMETER=3}
      mask prompts: FRAME_PERIOD "Period (ms)"; MAX_PARAMETER "Max counter"
      mask help:
      | Send a frame periodically or when an event parameter is updated.
      | - Period: Frame period (in ms)
      | Version : 01.00
      mask Help:
      | Send a frame periodically or when an event parameter is updated.
      | - Period: Frame period (in ms)
      | Version : 01.00
      mask Display:
      | disp('Mux Send Frame')
      | port_label('Input',1,'EVENT_DATA')
      | port_label('Output',1,'FRAME_SEND')
      | port_label('Output',2,'FRAME_PRESENT')

== system Renault_Library/Services ECU/PowerSupply ==
  note: PowerSupply
  RSA_PowerSupplyMgt : Subsystem  Tag=[LIB]  mask(RSA_PowerSupplyMgt){BattVoltageThresholdCalib=BatteryVoltage_ActivationThreshold_C, VoltageSupplyCutThresholdCalib=MemThreshold_C, WakeUpTimeCalib=WakeUpTime_C, ResetTimeCalib=ResetTime_C, SampleTimeCalib=SampleTime_C}
      mask prompts: BattVoltageThresholdCalib "Define the voltage threshold allowing activation of ECU"; VoltageSupplyCutThresholdCalib "Voltage threshold allowing cutting power with memorization. A voltage under this threshold cut immediately power supply of ECU."; WakeUpTimeCalib "Duration of Init phase"; ResetTimeCalib "Duration of Reset Phase"; SampleTimeCalib "SampleTime (not inherited)"
      mask help:
      | BatteryVoltage = voltage level of battery
      | (If no Battery Voltage is used, put a constant to 14Volt and set corresponding thresholds)
      | Apc = boolean to allow calculation, Key ON
      |
      | ActivateEcu = boolean to allow calculation taking into account the init/reset phases and Battery voltage
      | EcuStatus = variable giving the current status of Ecu (Off --> Init --> On --> Reset)
      | Read = flag which indicates the reading phase (during init or first step)
      | Write = flag which indicates the writing phase (during reset or powerlatch)
      |
      | Version : 01.01
      mask init:
      | Apc_ON=1;
      | Apc_OFF=0;
      | Ecu_ON=1;
      | Ecu_OFF=0;
      | 
      | Status_OFF=0;
      | Status_WAKEUP=2;
      | Status_ON=1;
      | Status_RESET=3;
      mask Help:
      | BatteryVoltage = voltage level of battery
      | (If no Battery Voltage is used, put a constant to 14Volt and set corresponding thresholds)
      | Apc = boolean to allow calculation, Key ON
      | 
      | ActivateEcu = boolean to allow calculation taking into account the init/reset phases and Battery voltage
      | EcuStatus = variable giving the current status of Ecu (Off --> Init --> On --> Reset)
      | Read = flag which indicates the reading phase (during init or first step)
      | Write = flag which indicates the writing phase (during reset or powerlatch)
      | 
      | Version : 01.01
      mask Display: disp('Power Supply Management')

== system Renault_Library/Standard blocks/Commonly Used Blocks ==
  note: Commonly Used Blocks
  In : Inport
  BusCreator1 : BusCreator  Inputs=2
  BusSelector1 : BusSelector  OutputSignals=signal1,signal2
  Constant1 : Constant  Value=ABC
  DataTypeConversion1 : DataTypeConversion  RndMeth=Zero
  Demux1 : Demux  Outputs=2
  LogicalOperator1 : Logic  OutDataTypeStr=boolean
  Mux1 : Mux  Inputs=2
  Product1 : Product  RndMeth=Zero
  RelationalOperator1 : RelationalOperator  Operator=<=  OutDataTypeStr=boolean  RndMeth=Nearest  InputSameDT=on
  Saturation1 : Saturate
  Subsystem : Subsystem  mask(Virtual Subsystem){}
      mask help: A subsystem block template containing an inport and outport block.
      settings: CopyFcn=set_param(gcbh,'LinkStatus','none'); set_param(gcbh,'Mask','off'); set_param(gcbh,'MaskType',''); set_param(gcbh,'MaskDescription',''); set_param(gcbh,'OpenFcn',''); set_param(gcbh,'CopyFcn','')  OpenFcn=open_system(gcbh,'force')
  Sum1 : Sum  OutDataTypeStr=Inherit: Inherit via back propagation  RndMeth=Zero  InputSameDT=on
      settings: AttributesFormatString=SAT = %<SaturateOnIntegerOverflow>
  Switch1 : Switch  Criteria=u2 ~= 0  RndMeth=Zero  InputSameDT=on
      settings: AttributesFormatString=Criteria=%<Criteria>
  Terminator1 : Terminator
  Out : Outport

== system Renault_Library/Standard blocks/Discontinuities ==
  note: /!\ DO NOT USE THIS RED BLOCKS ANYMORE /!\
  note: Discontinuities
  DeadZone : DeadZone  SaturateOnIntegerOverflow=on
  Quantizer : Quantizer
  RateLimiter : RateLimiter  SampleTimeMode=inherited
      settings: AttributesFormatString=Rising slew rate : %<RisingSlewLimit>\nFalling slew rate : %<FallingSlewLimit>
  Relay : Relay
  Saturation : Saturate
  SaturationDynamic : Subsystem  mask(Saturation Dynamic){OutMin=[], OutMax=[], OutDataTypeStr=Inherit: Same as second input, OutputDataTypeScalingMode=Same as second input, OutDataType=fixdt(1, 16), OutScaling=2^-10, LockScale=off, RndMeth=Zero, DoSatur=off}
      mask prompts: OutMin "Output minimum:"; OutMax "Output maximum:"; OutDataTypeStr "Output data type:"; OutputDataTypeScalingMode "Output data type mode:"; OutDataType "Output data type (e.g. fixdt(1, 16), fixdt('single')):"; OutScaling "Output scaling (Slope, e.g. 2^-9 or [Slope Bias], e.g. [1.25 3]):"; LockScale "Lock output data type setting against changes by the fixed-point tools"; RndMeth "Integer rounding mode:"; DoSatur "Saturate on integer overflow"
      mask help: Bound the range of the second input by using the first input (upper limit) and the third input (lower limit).
      mask init:
      | blkH = gcbh;
      | blkName = getfullname(blkH);
      | 
      | switch_blks = {'Switch','Switch2'}; 
      | for i = 1:length(switch_blks)
      |   set_param([blkName,'/',switch_blks{i}], ...
      |       'RndMeth', RndMeth, ...
      | 	  'DoSatur', DoSatur);
      | end
      mask Callback OutDataTypeStr: fixptdialog(gcbh)
      mask TypeOptions OutputDataTypeScalingMode: double | single | int8 | uint8 | int16 | uint16 | int32 | uint32 | Specify via dialog | Same as second input
      mask Callback OutputDataTypeScalingMode: fixptdialog(gcbh)
      mask Callback OutDataType: fixptdialog(gcbh)
      mask TypeOptions RndMeth: Ceiling | Convergent | Floor | Nearest | Round | Simplest | Zero
      mask Display: plot([0.15 0.85],[.5 .5],[.5 .5],[0.05 0.95],0.15+0.7*[0 .25 .75 1],0.05+0.9*[.25 .25 .75 .75]);
  Saturation_Dynamic : simulink/Discontinuities/Saturation Dynamic  SystemSampleTime=-1  OutMin=[]  OutMax=[]  OutDataTypeStr=Inherit: Same as second input  OutDataType=fixdt(1, 16)  OutScaling=2^-10  DoSatur=off

== system Renault_Library/Standard blocks/Discrete ==
  note: Discrete
  DiscreteStateSpace : DiscreteStateSpace  SampleTime=-1
  UnitDelay : UnitDelay  SampleTime=-1
      settings: AttributesFormatString=IC = %<X0>
